simulink model "ClimaCenCfgMgr"  (R2022b) (continued)
== system ClimaCenCfgMgr/ClimaCenCfgMgr_Runnable_sys == (continued)
      settings: EnsureOutportIsVirtual=on
  ClimaCfgForPostCdnAvl1_ClimaCfgForPostCdnAvl1_write : Outport  Port=123
      settings: EnsureOutportIsVirtual=on
  ClimaCfgForPreClng1_ClimaCfgForPreClng1_write : Outport  Port=124
      settings: EnsureOutportIsVirtual=on
  ClimaCfgForPrkgCDSNotAvl_ClimaCfgForPrkgCDSNotAvl_write : Outport  Port=125
      settings: EnsureOutportIsVirtual=on
  ClimaCfgForPrpsnTyp_ClimaCfgForPrpsnTyp_write : Outport  Port=126
      settings: EnsureOutportIsVirtual=on
  ClimaCfgForRoofHatch_ClimaCfgForRoofHatch_write : Outport  Port=127
      settings: EnsureOutportIsVirtual=on
  ClimaCfgForRowSecVentCentrVrnt1_ClimaCfgForRowSecVentCentrVrnt1_write : Outport  Port=128
      settings: EnsureOutportIsVirtual=on
  ClimaCfgForRowSecVentSidePrsnt1_ClimaCfgForRowSecVentSidePrsnt1_write : Outport  Port=129
      settings: EnsureOutportIsVirtual=on
  ClimaCfgForSeatHeatgCal1_ClimaCfgForSeatHeatgCal1_write : Outport  Port=130
      settings: EnsureOutportIsVirtual=on
  ClimaCfgForSeatHeatgForRowFirstPrsnt1_ClimaCfgForSeatHeatgForRowFirstPrsnt1_write : Outport  Port=131
      settings: EnsureOutportIsVirtual=on
  ClimaCfgForSeatHeatgForRowSec_ClimaCfgForSeatHeatgForRowSec_write : Outport  Port=132
      settings: EnsureOutportIsVirtual=on
  ClimaCfgForSeatHeatrElmDualPrsnt1_ClimaCfgForSeatHeatrElmDualPrsnt1_write : Outport  Port=133
      settings: EnsureOutportIsVirtual=on
  ClimaCfgForSeatVentnForRowFirst_ClimaCfgForSeatVentnForRowFirst_write : Outport  Port=134
      settings: EnsureOutportIsVirtual=on
  ClimaCfgForSeatVentnForRowSec_ClimaCfgForSeatVentnForRowSec_write : Outport  Port=135
      settings: EnsureOutportIsVirtual=on
  ClimaCfgForSmkSnsrPrsnt1_ClimaCfgForSmkSnsrPrsnt1_write : Outport  Port=136
      settings: EnsureOutportIsVirtual=on
  ClimaCfgForSnsrHumPrsnt1_ClimaCfgForSnsrHumPrsnt1_write : Outport  Port=137
      settings: EnsureOutportIsVirtual=on
  ClimaCfgForSnsrPrsntAtRowFirstAtFlrLe_ClimaCfgForSnsrPrsntAtRowFirstAtFlrLe_write : Outport  Port=138
      settings: EnsureOutportIsVirtual=on
  ClimaCfgForSnsrPrsntAtRowFirstAtFlrRi_ClimaCfgForSnsrPrsntAtRowFirstAtFlrRi_write : Outport  Port=139
      settings: EnsureOutportIsVirtual=on
  ClimaCfgForSnsrPrsntAtRowFirstAtVentSideLe_ClimaCfgForSnsrPrsntAtRowFirstAtVentSideLe_write : Outport  Port=140
      settings: EnsureOutportIsVirtual=on
  ClimaCfgForSnsrPrsntAtRowFirstAtVentSideRi_ClimaCfgForSnsrPrsntAtRowFirstAtVentSideRi_write : Outport  Port=141
      settings: EnsureOutportIsVirtual=on
  ClimaCfgForSnsrPrsntAtRowSecAtFlrLe_ClimaCfgForSnsrPrsntAtRowSecAtFlrLe_write : Outport  Port=142
      settings: EnsureOutportIsVirtual=on
  ClimaCfgForSnsrPrsntAtRowSecAtFlrRi_ClimaCfgForSnsrPrsntAtRowSecAtFlrRi_write : Outport  Port=143
      settings: EnsureOutportIsVirtual=on
  ClimaCfgForSnsrPrsntAtRowSecAtVentLe_ClimaCfgForSnsrPrsntAtRowSecAtVentLe_write : Outport  Port=144
      settings: EnsureOutportIsVirtual=on
  ClimaCfgForSnsrPrsntAtRowSecAtVentSideLe_ClimaCfgForSnsrPrsntAtRowSecAtVentSideLe_write : Outport  Port=145
      settings: EnsureOutportIsVirtual=on
  ClimaCfgForStatrtDevice_ClimaCfgForStartDevice_write : Outport  Port=146
      settings: EnsureOutportIsVirtual=on
  ClimaCfgForSteerWhlHeatgPrsnt1_ClimaCfgForSteerWhlHeatgPrsnt1_write : Outport  Port=147
      settings: EnsureOutportIsVirtual=on
  ClimaCfgForSteerWhlLeSide1_ClimaCfgForSteerWhlLeSide1_write : Outport  Port=148
      settings: EnsureOutportIsVirtual=on
  ClimaCfgForStrtStp_ClimaCfgForStrtStp_write : Outport  Port=149
      settings: EnsureOutportIsVirtual=on
  ClimaCfgForSunSnsr1_ClimaCfgForSunSnsr1_write : Outport  Port=150
      settings: EnsureOutportIsVirtual=on
  ClimaCfgForThermMgrPrsnt1_ClimaCfgForThermMgrPrsnt1_write : Outport  Port=151
      settings: EnsureOutportIsVirtual=on
  ClimaCfgForVehElec1_ClimaCfgForVehElec1_write : Outport  Port=152
      settings: EnsureOutportIsVirtual=on
  ClimaCfgForVehHev1_ClimaCfgForVehHev1_write : Outport  Port=153
      settings: EnsureOutportIsVirtual=on
  ClimaCfgForVehHyb1_ClimaCfgForVehHyb1_write : Outport  Port=154
      settings: EnsureOutportIsVirtual=on
  ClimaCfgForVehIce1_ClimaCfgForVehIce1_write : Outport  Port=155
      settings: EnsureOutportIsVirtual=on
  ClimaCfgForVehTyp_ClimaCfgForVehTyp_write : Outport  Port=156
      settings: EnsureOutportIsVirtual=on
  ClimaCfgForVgaMotorPrsntAtRowFirstLeMid_ClimaCfgForVgaMotorPrsntAtRowFirstLeMid_write : Outport  Port=157
      settings: EnsureOutportIsVirtual=on
  ClimaCfgForVgaMotorPrsntAtRowFirstRiMid_ClimaCfgForVgaMotorPrsntAtRowFirstRiMid_write : Outport  Port=158
      settings: EnsureOutportIsVirtual=on
  ClimaCfgForVgaMotorPrsntAtRowFirstSideLe_ClimaCfgForVgaMotorPrsntAtRowFirstSideLe_write : Outport  Port=159
      settings: EnsureOutportIsVirtual=on
  ClimaCfgForVgaMotorPrsntAtRowFirstSideRi_ClimaCfgForVgaMotorPrsntAtRowFirstSideRi_write : Outport  Port=160
      settings: EnsureOutportIsVirtual=on
  ClimaCfgForVgaMotorPrsntAtRowSecSideLe_ClimaCfgForVgaMotorPrsntAtRowSecSideLe_write : Outport  Port=161
      settings: EnsureOutportIsVirtual=on
  ClimaCfgForVgaMotorPrsntAtRowSecSideRi_ClimaCfgForVgaMotorPrsntAtRowSecSideRi_write : Outport  Port=162
      settings: EnsureOutportIsVirtual=on
  ClimaCfgForVrntOfVehSysElec1_ClimaCfgForVrntOfVehSysElec1_write : Outport  Port=163
      settings: EnsureOutportIsVirtual=on
  ClimaCfgForWindHeatrPrsnt1_ClimaCfgForWindHeatrPrsnt1_write : Outport  Port=164
      settings: EnsureOutportIsVirtual=on
  ClimaCfgForWtrPmpAuxPrsnt1_ClimaCfgForWtrPmpAuxPrsnt1_write : Outport  Port=165
      settings: EnsureOutportIsVirtual=on
  wires:
  CarCfgForClimaCtrl_CarCfgForAirClng_read/1 -> Terminator_1/1
  CarCfgForClimaCtrl_CarCfgForAirClnrAdv_read/1 -> Terminator_2/1
  CarCfgForClimaCtrl_CarCfgForAirFrag_read/1 -> Terminator_3/1
  CarCfgForClimaCtrl_CarCfgForAirMtrlSnsr_read/1 -> Terminator_4/1
  CarCfgForClimaCtrl_CarCfgForAirQly_read/1 -> Terminator_5/1
  CarCfgForClimaCtrl_CarCfgForAmntOfSeat_read/1 -> Terminator_6/1
  CarCfgForClimaCtrl_CarCfgForAutDemst_read/1 -> Terminator_7/1
  CarCfgForClimaCtrl_CarCfgForAuxWtrPmp_read/1 -> Terminator_8/1
  CarCfgForClimaCtrl_CarCfgForBypVlvInHvac_read/1 -> Terminator_9/1
  CarCfgForClimaCtrl_CarCfgForClsVlvInHvac_read/1 -> Terminator_10/1
  CarCfgForClimaCtrl_CarCfgForCmprAcTyp_read/1 -> Terminator_11/1
  CarCfgForClimaCtrl_CarCfgForCmptmtClng_read/1 -> Terminator_12/1
  CarCfgForClimaCtrl_CarCfgForElecVentAdjmt_read/1 -> Terminator_13/1
  CarCfgForClimaCtrl_CarCfgForExtVentnClimaCtrl_read/1 -> Terminator_14/1
  CarCfgForClimaCtrl_CarCfgForFlcTyp_read/1 -> Terminator_15/1
  CarCfgForClimaCtrl_CarCfgForFoldCSD_read/1 -> Terminator_16/1
  CarCfgForClimaCtrl_CarCfgForFuTyp_read/1 -> Terminator_17/1
  CarCfgForClimaCtrl_CarCfgForGlassTyp_read/1 -> Terminator_18/1
  CarCfgForClimaCtrl_CarCfgForHeadRadar_read/1 -> Terminator_19/1
  CarCfgForClimaCtrl_CarCfgForHeatPmp_read/1 -> Terminator_20/1
  CarCfgForClimaCtrl_CarCfgForHeatrDurgDrvg_read/1 -> Terminator_21/1
  CarCfgForClimaCtrl_CarCfgForHeatrTyp_read/1 -> Terminator_22/1
  CarCfgForClimaCtrl_CarCfgForHvacFilTyp_read/1 -> Terminator_23/1
  CarCfgForClimaCtrl_CarCfgForHvacFlrSnsrForT_read/1 -> Terminator_24/1
  CarCfgForClimaCtrl_CarCfgForHvacHeatrSnsrT_read/1 -> Terminator_25/1
  CarCfgForClimaCtrl_CarCfgForHvacRe_read/1 -> Terminator_26/1
  CarCfgForClimaCtrl_CarCfgForHvacTyp_read/1 -> Terminator_27/1
  CarCfgForClimaCtrl_CarCfgForLoVoltElecCfg_read/1 -> Terminator_28/1
  CarCfgForClimaCtrl_CarCfgForNavSysandIntnmap_read/1 -> Terminator_29/1
  CarCfgForClimaCtrl_CarCfgForNrOfDoor_read/1 -> Terminator_30/1
  CarCfgForClimaCtrl_CarCfgForPipeInFuTank_read/1 -> Terminator_31/1
  CarCfgForClimaCtrl_CarCfgForPreClng_read/1 -> Terminator_32/1
  CarCfgForClimaCtrl_CarCfgForPrkgCDSNotAvl_read/1 -> Terminator_33/1
  CarCfgForClimaCtrl_CarCfgForPrkgClima_read/1 -> Terminator_34/1
  CarCfgForClimaCtrl_CarCfgForPrpsnTyp_read/1 -> Terminator_35/1
  CarCfgForClimaCtrl_CarCfgForRemEngStrtPreCdn_read/1 -> Terminator_36/1
  CarCfgForClimaCtrl_CarCfgForRoofHatch_read/1 -> Terminator_37/1
  CarCfgForClimaCtrl_CarCfgForSeatHeatgCal_read/1 -> Terminator_38/1
  CarCfgForClimaCtrl_CarCfgForSeatHeatgForRowFirst_read/1 -> Terminator_39/1
  CarCfgForClimaCtrl_CarCfgForSeatHeatgForRowSec_read/1 -> Terminator_40/1
  CarCfgForClimaCtrl_CarCfgForSeatVentnForRowFirst_read/1 -> Terminator_41/1
  CarCfgForClimaCtrl_CarCfgForSeatVentnForRowSec_read/1 -> Terminator_42/1
  CarCfgForClimaCtrl_CarCfgForSecRowVentnCenter_read/1 -> Terminator_43/1
  CarCfgForClimaCtrl_CarCfgForSecRowVentnSide_read/1 -> Terminator_44/1
  CarCfgForClimaCtrl_CarCfgForSmallFlowCyc_read/1 -> Terminator_45/1
  CarCfgForClimaCtrl_CarCfgForSmkSnsr_read/1 -> Terminator_46/1
  CarCfgForClimaCtrl_CarCfgForSnsrForCmptmtT_read/1 -> Terminator_47/1
  CarCfgForClimaCtrl_CarCfgForSnsrHumPrsnt_read/1 -> Terminator_48/1
  CarCfgForClimaCtrl_CarCfgForStartDevice_read/1 -> Terminator_49/1
  CarCfgForClimaCtrl_CarCfgForSteerWhlHeatg_read/1 -> Terminator_50/1
  CarCfgForClimaCtrl_CarCfgForSteerWhlPosn_read/1 -> Terminator_51/1
  CarCfgForClimaCtrl_CarCfgForSunSnsr_read/1 -> Terminator_52/1
  CarCfgForClimaCtrl_CarCfgForTelmNodTyp_read/1 -> Terminator_53/1
  CarCfgForClimaCtrl_CarCfgForThermMngt_read/1 -> Terminator_54/1
  CarCfgForClimaCtrl_CarCfgForVehTyp_read/1 -> Terminator_55/1
  CarCfgForClimaCtrl_CarCfgForVgaMotorExistSts_read/1 -> Terminator_56/1
  CarCfgForClimaCtrl_CarCfgForWindHeatd_read/1 -> Terminator_57/1
  CooltVlvOutpTyp_CooltVlvOutpTyp_read/1 -> Terminator_58/1
  HvacCfgForClimaCtrl_HvacCfgForHvacFrontBlowerType_read/1 -> Terminator_59/1
  HvacCfgForClimaCtrl_HvacCfgForHvacMiscExistSts_read/1 -> Terminator_60/1
  HvacCfgForClimaCtrl_HvacCfgForHvacMotorExistSts_read/1 -> Terminator_61/1
  HvacCfgForClimaCtrl_HvacCfgForHvacSensorExistSts_read/1 -> Terminator_62/1
  CarCfgForClimaCtrl_CarCfgFor3RDROWSEATHEAT_read/1 -> Terminator_63/1
  CarCfgForClimaCtrl_CarCfgFor3RDROWSEATVENTILATED_read/1 -> Terminator_64/1
  CarCfgForClimaCtrl_CarCfgForBRAND_read/1 -> Terminator_65/1
  CarCfgForClimaCtrl_CarCfgForCabinOxgenSupply_read/1 -> Terminator_66/1
  CarCfgForClimaCtrl_CarCfgForElectricLiftingModule_read/1 -> Terminator_67/1
  CarCfgForClimaCtrl_CarCfgForFloatingDriving_read/1 -> Terminator_68/1
  CarCfgForClimaCtrl_CarCfgForInteriorViewCamera_read/1 -> Terminator_69/1
  CarCfgForClimaCtrl_CarCfgForLifeDetection_read/1 -> Terminator_70/1
  CarCfgForClimaCtrl_CarCfgForMARKET_read/1 -> Terminator_71/1
  CarCfgForClimaCtrl_CarCfgForPowerSeats_read/1 -> Terminator_72/1
  CarCfgForClimaCtrl_CarCfgForPTCInFrontHVAC_read/1 -> Terminator_73/1
  CarCfgForClimaCtrl_CarCfgForRFKEY_read/1 -> Terminator_74/1
  CarCfgForClimaCtrl_CarCfgForTEMPEREDSTORAGEBOX_read/1 -> Terminator_75/1
  CarCfgForClimaCtrl_CarCfgForVentLiftMotorExiSts_read/1 -> Terminator_76/1
  CarCfgForClimaCtrl_CarCfgForWadingAssistSystem_read/1 -> Terminator_77/1
  CarCfgForClimaCtrl_CarCfgWindFollowHumanMov_read/1 -> Terminator_78/1
  CenCfgMgrForCompile_CarCfgForAirClnrAdv_read/1 -> Terminator_79/1
  CenCfgMgrForCompile_CarCfgForAutDemst_read/1 -> Terminator_80/1
  CenCfgMgrForCompile_CarCfgForCmprAcTyp3_read/1 -> Terminator_81/1
  CenCfgMgrForCompile_CarCfgForHeatrTypGly_read/1 -> Terminator_82/1
  CenCfgMgrForCompile_CarCfgForSteerWhlHeatg_read/1 -> Terminator_83/1
  HvacCfgForClimaCtrl_HvacCfgForAQSTypes_read/1 -> Terminator_84/1
  HvacCfgForClimaCtrl_HvacCfgForHvacLowSideRETXVESts_read/1 -> Terminator_85/1
  HvacCfgForClimaCtrl_HvacCfgForHvacReBlowerType_read/1 -> Terminator_86/1
  HvacCfgForClimaCtrl_HvacCfgForHvacReVentPstn_read/1 -> Terminator_87/1
  HvacCfgForClimaCtrl_HvacCfgForHvacSnsrRCmptmtESts_read/1 -> Terminator_88/1
  HvacCfgForClimaCtrl_HvacCfgForHvacSnsrReHexESts_read/1 -> Terminator_89/1
  HvacCfgReqVersionReplace_HvacCfgReqVersionReplace_read/1 -> Terminator_90/1
  Ground_1/1 -> ClimaCfgFor3RDROWSEATHEAT_ClimaCfgFor3RDROWSEATHEAT_write/1
  Ground_2/1 -> ClimaCfgFor3RDROWSEATVENTILATED_ClimaCfgFor3RDROWSEATVENTILATED_write/1
  Ground_3/1 -> ClimaCfgForAAC_ClimaCfgForAAC_write/1
  Ground_4/1 -> ClimaCfgForAQSTypes_ClimaCfgForAQSTypes_write/1
  Ground_5/1 -> ClimaCfgForAutDemst_ClimaCfgForAutDemst_write/1
  Ground_6/1 -> ClimaCfgForBRAND_ClimaCfgForBRAND_write/1
  Ground_7/1 -> ClimaCfgForCabinOxgenSupply_ClimaCfgForCabinOxgenSupply_write/1
  Ground_8/1 -> ClimaCfgForDrvrSeatVentWthSmf2_ClimaCfgForDrvrSeatVentWthSmf2_write/1
  Ground_9/1 -> ClimaCfgForElectricLiftingModule_ClimaCfgForElectricLiftingModule_write/1
  Ground_10/1 -> ClimaCfgForErev1_ClimaCfgForErev1_write/1
  Ground_11/1 -> ClimaCfgForFLOATINGDRVING_ClimaCfgForFLOATINGDRVING_write/1
  Ground_12/1 -> ClimaCfgForHvacLowSideRETXVESts_ClimaCfgForHvacLowSideRETXVESts_write/1
  Ground_13/1 -> ClimaCfgForHvacReBlowerType_ClimaCfgForHvacReBlowerType_write/1
  Ground_14/1 -> ClimaCfgForHvacReTmpZn_ClimaCfgForHvacReTmpZn_write/1
  Ground_15/1 -> ClimaCfgForHvacReVentPstn_ClimaCfgForRowSecAtFlrLeRe_write/1
  Ground_16/1 -> ClimaCfgForHvacReVentPstn_ClimaCfgForRowSecAtFlrRiRe_write/1
  Ground_17/1 -> ClimaCfgForHvacReVentPstn_ClimaCfgForRowSecAtVentSideTopLe_write/1
  Ground_18/1 -> ClimaCfgForHvacReVentPstn_ClimaCfgForRowSecAtVentSideTopRi_write/1
  Ground_19/1 -> ClimaCfgForHvacReVentPstn_ClimaCfgForRowThrdAtFlrLe_write/1
  Ground_20/1 -> ClimaCfgForHvacReVentPstn_ClimaCfgForRowThrdAtFlrRi_write/1
  Ground_21/1 -> ClimaCfgForHvacReVentPstn_ClimaCfgForRowThrdAtVentSideTopLe_write/1
  Ground_22/1 -> ClimaCfgForHvacReVentPstn_ClimaCfgForRowThrdAtVentSideTopRi_write/1
  Ground_23/1 -> ClimaCfgForHvacSnsrRCmptmtESts_ClimaCfgForHvacSnsrRCmptmtESts_write/1
  Ground_24/1 -> ClimaCfgForHvacSnsrReHexESts_ClimaCfgForHvacSnsrReHexESts_write/1
  Ground_25/1 -> ClimaCfgForHvacTyp_ClimaCfgForHvacTyp_write/1
  Ground_26/1 -> ClimaCfgForInteriorViewCamera_ClimaCfgForInteriorViewCamera_write/1
  Ground_27/1 -> ClimaCfgForLifeDetection_ClimaCfgForLifeDetection_write/1
  Ground_28/1 -> ClimaCfgForMARKET_ClimaCfgForMARKET_write/1
  Ground_29/1 -> ClimaCfgForPassSeatVentWthSmf2_ClimaCfgForPassSeatVentWthSmf2_write/1
  Ground_30/1 -> ClimaCfgForPowerSeats_ClimaCfgForPowerSeats_write/1
  Ground_31/1 -> ClimaCfgForPTCInFrontHVAC_ClimaCfgForPTCInFrontHVAC_write/1
  Ground_32/1 -> ClimaCfgForRFKEY_ClimaCfgForRFKEY_write/1
  Ground_33/1 -> ClimaCfgForTEMPEREDSTORAGEBOX_ClimaCfgForTEMPEREDSTORAGEBOX_write/1
  Ground_34/1 -> ClimaCfgForVgaMotor1_ClimaCfgForVgaMotor1_write/1
  Ground_35/1 -> ClimaCfgForVgaMotor10_ClimaCfgForVgaMotor10_write/1
  Ground_36/1 -> ClimaCfgForVgaMotor11_ClimaCfgForVgaMotor11_write/1
  Ground_37/1 -> ClimaCfgForVgaMotor12_ClimaCfgForVgaMotor12_write/1
  Ground_38/1 -> ClimaCfgForVgaMotor13_ClimaCfgForVgaMotor13_write/1
  Ground_39/1 -> ClimaCfgForVgaMotor14_ClimaCfgForVgaMotor14_write/1
  Ground_40/1 -> ClimaCfgForVgaMotor15_ClimaCfgForVgaMotor15_write/1
  Ground_41/1 -> ClimaCfgForVgaMotor16_ClimaCfgForVgaMotor16_write/1
  Ground_42/1 -> ClimaCfgForVgaMotor17_ClimaCfgForVgaMotor17_write/1
  Ground_43/1 -> ClimaCfgForVgaMotor18_ClimaCfgForVgaMotor18_write/1
  Ground_44/1 -> ClimaCfgForVgaMotor19_ClimaCfgForVgaMotor19_write/1
  Ground_45/1 -> ClimaCfgForVgaMotor2_ClimaCfgForVgaMotor2_write/1
  Ground_46/1 -> ClimaCfgForVgaMotor20_ClimaCfgForVgaMotor20_write/1
  Ground_47/1 -> ClimaCfgForVgaMotor3_ClimaCfgForVgaMotor3_write/1
  Ground_48/1 -> ClimaCfgForVgaMotor4_ClimaCfgForVgaMotor4_write/1
  Ground_49/1 -> ClimaCfgForVgaMotor5_ClimaCfgForVgaMotor5_write/1
  Ground_50/1 -> ClimaCfgForVgaMotor6_ClimaCfgForVgaMotor6_write/1
  Ground_51/1 -> ClimaCfgForVgaMotor7_ClimaCfgForVgaMotor7_write/1
  Ground_52/1 -> ClimaCfgForVgaMotor8_ClimaCfgForVgaMotor8_write/1
  Ground_53/1 -> ClimaCfgForVgaMotor9_ClimaCfgForVgaMotor9_write/1
  Ground_54/1 -> ClimaCfgForWadingAssistSystem_ClimaCfgForWadingAssistSystem_write/1
  Ground_55/1 -> ClimaCfgHvacForFrntLeTempActr_ClimaCfgHvacForFrntLeTempActr_write/1
  Ground_56/1 -> ClimaCfgHvacForFrntRiTempActr_ClimaCfgHvacForFrntRiTempActr_write/1
  Ground_57/1 -> ClimaCfgHvacFrntForRowSecForActrTLe_ClimaCfgHvacFrntForRowSecForActrTLe_write/1
  Ground_58/1 -> ClimaCfgHvacFrntForRowSecForActrTRi_ClimaCfgHvacFrntForRowSecForActrTRi_write/1
  Ground_59/1 -> ClimaCfgHvacReForRowSecForActrTLe_ClimaCfgHvacReForRowSecForActrTLe_write/1
  Ground_60/1 -> ClimaCfgHvacReForRowSecForActrTRi_ClimaCfgHvacReForRowSecForActrTRi_write/1
  Ground_61/1 -> ClimaCfgReqVersionReplace_ClimaCfgReqVersionReplace_write/1
  Ground_62/1 -> ClimaCfgWindFollowHumanMov_ClimaCfgWindFollowHumanMov_write/1
  Ground_63/1 -> ClimaCfgForActrModVrnt1_ClimaCfgForActrInd1_write/1
  Ground_64/1 -> ClimaCfgForActrModVrnt1_ClimaCfgForActrModVrnt1_write/1
  Ground_65/1 -> ClimaCfgForActrModVrnt1_ClimaCfgForActrModVrntCen_write/1
  Ground_66/1 -> ClimaCfgForActrModVrnt2_ClimaCfgForActrInd2_write/1
  Ground_67/1 -> ClimaCfgForActrModVrnt2_ClimaCfgForActrModVrnt2_write/1
  Ground_68/1 -> ClimaCfgForActrRecircAndOsaCmnPrsnt1_ClimaCfgForActrRecircAndOsaCmnPrsnt1_write/1
  Ground_69/1 -> ClimaCfgForAirClng1_ClimaCfgForAirClng1_write/1
  Ground_70/1 -> ClimaCfgForAirClnrAdv1_ClimaCfgForAirClnrAdv1_write/1
  Ground_71/1 -> ClimaCfgForAirFrag_ClimaCfgForAirFrag_write/1
  Ground_72/1 -> ClimaCfgForAirMtrlSnsrAPMAPrsnt1_ClimaCfgForAirMtrlSnsrAPMAPrsnt1_write/1
  Ground_73/1 -> ClimaCfgForAirMtrlSnsrPrsnt1_ClimaCfgForAirMtrlSnsrPrsnt1_write/1
  Ground_74/1 -> ClimaCfgForAirQly1_ClimaCfgForAirQly1_write/1
  Ground_75/1 -> ClimaCfgForAmntOfSeat1_ClimaCfgForAmntOfSeat1_write/1
  Ground_76/1 -> ClimaCfgForAmntOfSeatRow1_ClimaCfgForAmntOfSeatRow1_write/1
  Ground_77/1 -> ClimaCfgForBlowrInRowSecVentSidePrsnt1_ClimaCfgForBlowrInRowSecVentSidePrsnt1_write/1
  Ground_78/1 -> ClimaCfgForBypVlvInHvacExtCtrl1_ClimaCfgForBypVlvInHvacExtCtrl1_write/1
  Ground_79/1 -> ClimaCfgForBypVlvInHvacPrsnt1_ClimaCfgForBypVlvInHvacPrsnt1_write/1
  Ground_80/1 -> ClimaCfgForCarTyp1_ClimaCfgForCarTyp1_write/1
  Ground_81/1 -> ClimaCfgForCds1_ClimaCfgForCds1_write/1
  Ground_82/1 -> ClimaCfgForClimaCtrlMan1_ClimaCfgForClimaCtrlMan1_write/1
  Ground_83/1 -> ClimaCfgForClimaCtrlSwtModlPrsnt_ClimaCfgForClimaCtrlSwtModlPrsnt_write/1
  Ground_84/1 -> ClimaCfgForCmptmtClng_ClimaCfgForCmptmtClng_write/1
  Ground_85/1 -> ClimaCfgForCmptmtSnsr1_ClimaCfgForCmptmtSnsr1_write/1
  Ground_86/1 -> ClimaCfgForComIfGenn2ForTelm1_ClimaCfgForComIfGenn2ForTelm1_write/1
  Ground_87/1 -> ClimaCfgForCoolgPreCdn1_ClimaCfgForCoolgPreCdn1_write/1
  Ground_88/1 -> ClimaCfgForCooltCircForClima1_ClimaCfgForCooltCircForClima1_write/1
  Ground_89/1 -> ClimaCfgForCpilHeatrElecPrsnt1_ClimaCfgForCpilHeatrElecPrsnt1_write/1
  Ground_90/1 -> ClimaCfgForEcuCooltVlvOutp1_ClimaCfgForEcuCooltVlvOutp1_write/1
  Ground_91/1 -> ClimaCfgForEngCooltPmpElec1_ClimaCfgForEngCooltPmpElec1_write/1
  Ground_92/1 -> ClimaCfgForExtVentnClimaCtrl_ClimaCfgForExtVentnClimaCtrl_write/1
  Ground_93/1 -> ClimaCfgForFanPotiCtrld1_ClimaCfgForFanPotiCtrld1_write/1
  Ground_94/1 -> ClimaCfgForFlcTyp_ClimaCfgForFlcTyp_write/1
  Ground_95/1 -> ClimaCfgForFlrSnsrInHvac1_ClimaCfgForFlrSnsrInHvac1_write/1
  Ground_96/1 -> ClimaCfgForFoldCSD_ClimaCfgForFoldCSD_write/1
  Ground_97/1 -> ClimaCfgForForElecVentAdjmt_ClimaCfgForForElecVentAdjmt_write/1
  Ground_98/1 -> ClimaCfgForForHeatPmp_ClimaCfgForForHeatPmp_write/1
  Ground_99/1 -> ClimaCfgForForHeatPmpGly_ClimaCfgForForHeatPmp_write/1
  Ground_100/1 -> ClimaCfgForForLoVoltElecCfg_ClimaCfgForForLoVoltElecCfg_write/1
  Ground_101/1 -> ClimaCfgForForRemEngStrtPreCdn_ClimaCfgForForRemEngStrtPreCdn_write/1
  Ground_102/1 -> ClimaCfgForFuHeatrFuLine1_ClimaCfgForFuHeatrFuLine1_write/1
  Ground_103/1 -> ClimaCfgForFuHeatrTyp1_ClimaCfgForFuHeatrTyp1_write/1
  Ground_104/1 -> ClimaCfgForGlassTyp1_ClimaCfgForGlassTyp1_write/1
  Ground_105/1 -> ClimaCfgForHeadRadar_ClimaCfgForHeadRadar_write/1
  Ground_106/1 -> ClimaCfgForHeatgPreCdnAvl1_ClimaCfgForHeatgPreCdnAvl1_write/1
  Ground_107/1 -> ClimaCfgForHeatrTyp1_ClimaCfgForHeatrTyp1_write/1
  Ground_108/1 -> ClimaCfgForHvacFilVrnt1_ClimaCfgForHvacFilVrnt1_write/1
  Ground_109/1 -> ClimaCfgForHvacFrontBlowerType_ClimaCfgForHvacFrontBlowerType_write/1
  Ground_110/1 -> ClimaCfgForHvacHeatrElecFrntPrsnt1_ClimaCfgForHvacHeatrElecFrntPrsnt1_write/1
  Ground_111/1 -> ClimaCfgForHvacHeatrSnsrTPrsnt1_ClimaCfgForHvacHeatrSnsrTPrsnt1_write/1
  Ground_112/1 -> ClimaCfgForHvacManWithPartRecirc2_ClimaCfgForHvacManWithPartRecirc2_write/1
  Ground_113/1 -> ClimaCfgForHvacMiscExistSts_ClimaCfgForHvacMiscExistSts_write/1
  Ground_114/1 -> ClimaCfgForHvacRePrsnt1_ClimaCfgForHvacRePrsnt1_write/1
  Ground_115/1 -> ClimaCfgForHvacReTyp1_ClimaCfgForHvacReTyp1_write/1
  Ground_116/1(Out1) -> ClimaCfgForHvacSnsrCfg1_ClimaCfgForHvacSnsrCfg1_write/1
  Ground_117/1 -> ClimaCfgForHvHeatrTyp1_ClimaCfgForHvHeatrTyp1_write/1
  Ground_118/1 -> ClimaCfgForNavSysandIntnmap_ClimaCfgForNavSysandIntnmap_write/1
  Ground_119/1 -> ClimaCfgForNrOfAirDistbnSetg1_ClimaCfgForNrOfAirDistbnSetg1_write/1
  Ground_120/1 -> ClimaCfgForNrOfDoor1_ClimaCfgForNrOfDoor1_write/1
  Ground_121/1 -> ClimaCfgForNrOfFanLvlSetg1_ClimaCfgForNrOfFanLvlSetg1_write/1
  Ground_122/1 -> ClimaCfgForNrOfTSetg1_ClimaCfgForNrOfTSetg1_write/1
  Ground_123/1 -> ClimaCfgForPostCdnAvl1_ClimaCfgForPostCdnAvl1_write/1
  Ground_124/1 -> ClimaCfgForPreClng1_ClimaCfgForPreClng1_write/1
  Ground_125/1 -> ClimaCfgForPrkgCDSNotAvl_ClimaCfgForPrkgCDSNotAvl_write/1
  Ground_126/1 -> ClimaCfgForPrpsnTyp_ClimaCfgForPrpsnTyp_write/1
  Ground_127/1 -> ClimaCfgForRoofHatch_ClimaCfgForRoofHatch_write/1
  Ground_128/1 -> ClimaCfgForRowSecVentCentrVrnt1_ClimaCfgForRowSecVentCentrVrnt1_write/1
  Ground_129/1 -> ClimaCfgForRowSecVentSidePrsnt1_ClimaCfgForRowSecVentSidePrsnt1_write/1
  Ground_130/1 -> ClimaCfgForSeatHeatgCal1_ClimaCfgForSeatHeatgCal1_write/1
  Ground_131/1 -> ClimaCfgForSeatHeatgForRowFirstPrsnt1_ClimaCfgForSeatHeatgForRowFirstPrsnt1_write/1
  Ground_132/1 -> ClimaCfgForSeatHeatgForRowSec_ClimaCfgForSeatHeatgForRowSec_write/1
  Ground_133/1 -> ClimaCfgForSeatHeatrElmDualPrsnt1_ClimaCfgForSeatHeatrElmDualPrsnt1_write/1
  Ground_134/1 -> ClimaCfgForSeatVentnForRowFirst_ClimaCfgForSeatVentnForRowFirst_write/1
  Ground_135/1 -> ClimaCfgForSeatVentnForRowSec_ClimaCfgForSeatVentnForRowSec_write/1
  Ground_136/1 -> ClimaCfgForSmkSnsrPrsnt1_ClimaCfgForSmkSnsrPrsnt1_write/1
  Ground_137/1 -> ClimaCfgForSnsrHumPrsnt1_ClimaCfgForSnsrHumPrsnt1_write/1
  Ground_138/1 -> ClimaCfgForSnsrPrsntAtRowFirstAtFlrLe_ClimaCfgForSnsrPrsntAtRowFirstAtFlrLe_write/1
  Ground_139/1 -> ClimaCfgForSnsrPrsntAtRowFirstAtFlrRi_ClimaCfgForSnsrPrsntAtRowFirstAtFlrRi_write/1
  Ground_140/1 -> ClimaCfgForSnsrPrsntAtRowFirstAtVentSideLe_ClimaCfgForSnsrPrsntAtRowFirstAtVentSideLe_write/1
  Ground_141/1 -> ClimaCfgForSnsrPrsntAtRowFirstAtVentSideRi_ClimaCfgForSnsrPrsntAtRowFirstAtVentSideRi_write/1
  Ground_142/1 -> ClimaCfgForSnsrPrsntAtRowSecAtFlrLe_ClimaCfgForSnsrPrsntAtRowSecAtFlrLe_write/1
  Ground_143/1 -> ClimaCfgForSnsrPrsntAtRowSecAtFlrRi_ClimaCfgForSnsrPrsntAtRowSecAtFlrRi_write/1
  Ground_144/1 -> ClimaCfgForSnsrPrsntAtRowSecAtVentLe_ClimaCfgForSnsrPrsntAtRowSecAtVentLe_write/1
  Ground_145/1 -> ClimaCfgForSnsrPrsntAtRowSecAtVentSideLe_ClimaCfgForSnsrPrsntAtRowSecAtVentSideLe_write/1
  Ground_146/1 -> ClimaCfgForStatrtDevice_ClimaCfgForStartDevice_write/1
  Ground_147/1 -> ClimaCfgForSteerWhlHeatgPrsnt1_ClimaCfgForSteerWhlHeatgPrsnt1_write/1
  Ground_148/1 -> ClimaCfgForSteerWhlLeSide1_ClimaCfgForSteerWhlLeSide1_write/1
  Ground_149/1 -> ClimaCfgForStrtStp_ClimaCfgForStrtStp_write/1
  Ground_150/1 -> ClimaCfgForSunSnsr1_ClimaCfgForSunSnsr1_write/1
  Ground_151/1 -> ClimaCfgForThermMgrPrsnt1_ClimaCfgForThermMgrPrsnt1_write/1
  Ground_152/1 -> ClimaCfgForVehElec1_ClimaCfgForVehElec1_write/1
  Ground_153/1 -> ClimaCfgForVehHev1_ClimaCfgForVehHev1_write/1
  Ground_154/1 -> ClimaCfgForVehHyb1_ClimaCfgForVehHyb1_write/1
  Ground_155/1 -> ClimaCfgForVehIce1_ClimaCfgForVehIce1_write/1
  Ground_156/1 -> ClimaCfgForVehTyp_ClimaCfgForVehTyp_write/1
  Ground_157/1 -> ClimaCfgForVgaMotorPrsntAtRowFirstLeMid_ClimaCfgForVgaMotorPrsntAtRowFirstLeMid_write/1
  Ground_158/1 -> ClimaCfgForVgaMotorPrsntAtRowFirstRiMid_ClimaCfgForVgaMotorPrsntAtRowFirstRiMid_write/1
  Ground_159/1 -> ClimaCfgForVgaMotorPrsntAtRowFirstSideLe_ClimaCfgForVgaMotorPrsntAtRowFirstSideLe_write/1
  Ground_160/1 -> ClimaCfgForVgaMotorPrsntAtRowFirstSideRi_ClimaCfgForVgaMotorPrsntAtRowFirstSideRi_write/1
  Ground_161/1 -> ClimaCfgForVgaMotorPrsntAtRowSecSideLe_ClimaCfgForVgaMotorPrsntAtRowSecSideLe_write/1
  Ground_162/1 -> ClimaCfgForVgaMotorPrsntAtRowSecSideRi_ClimaCfgForVgaMotorPrsntAtRowSecSideRi_write/1
  Ground_163/1 -> ClimaCfgForVrntOfVehSysElec1_ClimaCfgForVrntOfVehSysElec1_write/1
  Ground_164/1 -> ClimaCfgForWindHeatrPrsnt1_ClimaCfgForWindHeatrPrsnt1_write/1
  Ground_165/1 -> ClimaCfgForWtrPmpAuxPrsnt1_ClimaCfgForWtrPmpAuxPrsnt1_write/1

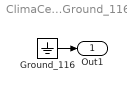
== system ClimaCenCfgMgr/ClimaCenCfgMgr_Runnable_sys/Ground_116 ==
  Ground_116 : Ground
  Out1 : Outport  OutDataTypeStr=Bus: HvacSnsrCfg1  PortDimensions=1
  wires:
  Ground_116/1 -> Out1/1
